simulink model "ofdm_txrx_mimo"  (Simulink 7.1)
settings: Solver=ode45  StartTime=0.0  StopTime=6e3  RelTol=1e-3
PreLoadFcn:
  | ofdm_rx_mimo_init
InitFcn:
  | ofdm_rx_mimo_init
StartFcn:
  | ofdm_rx_mimo_init
values from neighbouring files (symbols this model uses):
  BER_RAM_init_size = 2048    [ofdm_tx_mimo_init.m]
  CPLength = 16    [ofdm_tx_mimo_init.m]
  CRCPolynomial = hex2dec('04c11db7')    [ofdm_tx_mimo_init.m]
  freqOffset_track_filtCoef_i = hex2dec('2500')/2^32    [ofdm_rx_mimo_init.m]
  freqOffset_track_filtCoef_p = hex2dec('d0000')/2^32    [ofdm_rx_mimo_init.m]
  hi = zeros(1,length(cci))    [ofdm_rx_mimo_init.m]
  hr = zeros(1,length(ccr))    [ofdm_rx_mimo_init.m]
  max_CP_length = 16    [ofdm_tx_mimo_init.m]
  max_num_baseRateSymbols = 31    [ofdm_tx_mimo_init.m]
  max_num_subcarriers = 64    [ofdm_tx_mimo_init.m]
  max_num_trainingSymbols = 15    [ofdm_tx_mimo_init.m]
  max_numBytes = 16384    [ofdm_tx_mimo_init.m]
  max_OFDM_symbols = 2047    [ofdm_tx_mimo_init.m]
  modConstellation_qam16 = .75*[1 3 -1 -3]./3    [ofdm_tx_mimo_init.m]
  modConstellation_qam256 = 0.9375*[3 1 5 7 11 9 13 15 -3 -1 -5 -7 -11 -9 -13 -15]./15    [ofdm_tx_mimo_init.m]
  modConstellation_qam64 = 0.875*[3 1 5 7 -3 -1 -5 -7]./7    [ofdm_tx_mimo_init.m]
  modConstellation_qpsk = [1 -1]./sqrt(2)    [ofdm_tx_mimo_init.m]
  numBaseRateSymbols = 2    [ofdm_tx_mimo_init.m]
  numSubcarriers = 64    [ofdm_tx_mimo_init.m]
  numTrainingSymbols = 2    [ofdm_tx_mimo_init.m]
  pilot1_indicies = 7 + ( (64-7) * 2^16)    (= 3735559)    [ofdm_tx_mimo_init.m]
  pilot2_indicies = 21 + ( (64-21) * 2^16)    (= 2818069)    [ofdm_tx_mimo_init.m]
  pilotValue_neg = hex2dec('8000') + (2^16 * 0)    [ofdm_tx_mimo_init.m]
  pilotValue_pos = hex2dec('7FFF') + (2^16 * 0)    [ofdm_tx_mimo_init.m]
  pkt_crossCorr_thresh = 0.7    [ofdm_rx_mimo_init.m]
  pkt_energy_thresh = 0    [ofdm_rx_mimo_init.m]
  pkt_numPayloadBytes = 1400+42-6    (= 1436)    [ofdm_tx_mimo_init.m]
  pktByteNums = numHeaderBytes +  				byteIndex_numPayloadBytes(1)*2^8 +  				byteIndex_numPayloadBytes(2)*2^16 +  				byteIndex_simpleDynModMasks * 2^24    [ofdm_rx_mimo_init.m]
  PktDet_Delay = 26 + Symbol_Timing_Offset*2^7    (= 1050)    [ofdm_rx_mimo_init.m]
  preamble = 6*[0 shortsyms_10 longsyms_2]    [ofdm_tx_mimo_init.m]
  preamble_I = real(preamble)    [ofdm_tx_mimo_init.m]
  preamble_Q = imag(preamble)    [ofdm_tx_mimo_init.m]
  RAM_init_size = max_numBytes    (= 16384)    [ofdm_tx_mimo_init.m]
  reg_Interrupt_PktBuf_Control = reg_InterruptControl +  	1 * 2^16 +  	0 * 2^24    (= 65656)    [ofdm_rx_mimo_init.m]
  rx_controlBits = 1 * 2  		 + 1 * 4  		 + 0 * 8  		 + 1 * 16 * rx_SISO_Mode  		 + 1 * 32  		 + 1 * 64  		 + 0 * 128  		 + 1 * 256  		 + 0 * 512  		 + 1 * 1024  		 + 0 * 2048  		 + 1 * 4096  		 + 1 * 8192  		 + 0 * 16384  		 + 1 * 65536  		 + 1 * 131072  		 + 0 * 262144  		 + 1 * 524288    [ofdm_rx_mimo_init.m]
  Rx_PhaseNoiseTrack_K = 0.6094    [ofdm_rx_mimo_init.m]
  rx_postEq_scaling = round(rxScaling*2^11) * (1 + 2^16)    [ofdm_rx_mimo_init.m]
  RxDataScrambling_Seq = [40 198 78 63 82 173 102 245 48 111 172 115 147 230 216 93 72 65 62 2 205 242 122 90 128 83 105 97 73 10 5 252]    [ofdm_tx_mimo_init.m]
  simOnly_numSamples = length(preamble)+( (numSubcarriers+CPLength)*(numTrainingSymbols + numBaseRateSymbols + numFullRateSymbols/2) )    [ofdm_tx_mimo_init.m]
  train = [train train]    [ofdm_tx_mimo_init.m]
  tx_controlBits = (antB_preambleShift * 2^4) + (2^0 * tx_SISO_Mode) + 4*0 + 8*1    (= 73)    [ofdm_tx_mimo_init.m]
  TxDataScrambling_Seq = [40 198 78 63 82 173 102 245 48 111 172 115 147 230 216 93 72 65 62 2 205 242 122 90 128 83 105 97 73 10 5 252]    [ofdm_tx_mimo_init.m]
  txReg_symbolCounts = (2^16 * numFullRateSymbols) + (2^8 *numBaseRateSymbols ) + numTrainingSymbols    [ofdm_tx_mimo_init.m]
  TxRx_FFTScaling = bin2dec('010111') + (bin2dec('000101') * 2^6)    [ofdm_tx_mimo_init.m]
undefined symbols (not defined in the file or in its folder): AntA, AntB, BaseRate, CRC_table_gen, gcbh, intfilt, pktTiming_count_payloadStart, pktTiming_symbol_length, xlBlockLoadCallback, xlGetSimulinkPeriod
libraries: xbsIndex_r4 x4212, dspmlti4 x4, dspsigops x4, commchan3 x2, simulink x2, commrflib2 x2, dspsrcs4 x1
(part 21 of 43)

== system ofdm_txrx_mimo/OFDM Rx MIMO/Long Corr 4x/C7/C11 ==
  xn : Inport  OutDataType=sfix(16)  OutScaling=2^0
  DAddr : Inport  Port=2  OutDataType=sfix(16)  OutScaling=2^0
  CAddr : Inport  Port=3  OutDataType=sfix(16)  OutScaling=2^0
  LD : Inport  Port=4  OutDataType=sfix(16)  OutScaling=2^0
  Down Sample1 : xbsIndex_r4/Down Sample  sample_ratio=length(coef2)  sample_phase=First Value of Frame  en=off  latency=0  dbl_ovrd=off  block_type=dsamp
      settings: xl_area=[3 3 0 2 0 0 0]  block_version=VER_STRING_GOES_HERE
  Down Sample2 : xbsIndex_r4/Down Sample  sample_ratio=length(coef2)  sample_phase=First Value of Frame  en=off  latency=0  dbl_ovrd=off  block_type=dsamp
      settings: xl_area=[5 5 0 4 0 0 0]  block_version=VER_STRING_GOES_HERE
  MAC : Subsystem
  R : xbsIndex_r4/Addressable Shift Register  infer_latency=off  depth=length(coef2)  initVector=[0]  en=off  dbl_ovrd=off  use_behavioral_HDL=off  use_rpm=off  block_type=addrsr
      settings: infoedit="Delay of  configurable length.  Any element  in the delay     line   can    be   addressed   and   driven    onto   the   output     port.<P><P>Hardware  notes: Implemented  using SRL16s.     If Virtex-4, Virtex-II or Spartan-3 devices are used, multiple SRLC16s are cascaded together."  xl_area=[1 0 0 2 0 0 0]  block_version=VER_STRING_GOES_HERE
  ROM1 : xbsIndex_r4/ROM  depth=length(coef2)  initVector=coef2  distributed_mem=Distributed memory  rst=off  init_reg=0  en=off  latency=1  arith_type=Unsigned  n_bits=1  bin_pt=0  dbl_ovrd=off  optimize=Area  use_rpm=off  block_type=sprom
      settings: xl_area=[1 1 0 1 0 0 0]  block_version=VER_STRING_GOES_HERE
  yn : Outport  OutDataType=sfix(16)  OutScaling=2^0
  xnz : Outport  Port=2  OutDataType=sfix(16)  OutScaling=2^0
  wires:
  Down Sample1/1 -> R/1
  xn/1 -> Down Sample1/1
  DAddr/1 -> R/2
  CAddr/1 -> ROM1/1
  R/1 -> xnz/1, MAC/1(a)
  MAC/1(yn) -> Down Sample2/1
  Down Sample2/1 -> yn/1
  ROM1/1 -> MAC/2(coeff)
  LD/1 -> MAC/3(ld)

== system ofdm_txrx_mimo/OFDM Rx MIMO/Long Corr 4x/C7/C12 ==
  xn : Inport  OutDataType=sfix(16)  OutScaling=2^0
  DAddr : Inport  Port=2  OutDataType=sfix(16)  OutScaling=2^0
  CAddr : Inport  Port=3  OutDataType=sfix(16)  OutScaling=2^0
  LD : Inport  Port=4  OutDataType=sfix(16)  OutScaling=2^0
  Down Sample1 : xbsIndex_r4/Down Sample  sample_ratio=length(coef2)  sample_phase=First Value of Frame  en=off  latency=0  dbl_ovrd=off  block_type=dsamp
      settings: xl_area=[3 3 0 2 0 0 0]  block_version=VER_STRING_GOES_HERE
  Down Sample2 : xbsIndex_r4/Down Sample  sample_ratio=length(coef2)  sample_phase=First Value of Frame  en=off  latency=0  dbl_ovrd=off  block_type=dsamp
      settings: xl_area=[5 5 0 4 0 0 0]  block_version=VER_STRING_GOES_HERE
  MAC : Subsystem
  R : xbsIndex_r4/Addressable Shift Register  infer_latency=off  depth=length(coef2)  initVector=[0]  en=off  dbl_ovrd=off  use_behavioral_HDL=off  use_rpm=off  block_type=addrsr
      settings: infoedit="Delay of  configurable length.  Any element  in the delay     line   can    be   addressed   and   driven    onto   the   output     port.<P><P>Hardware  notes: Implemented  using SRL16s.     If Virtex-4, Virtex-II or Spartan-3 devices are used, multiple SRLC16s are cascaded together."  xl_area=[1 0 0 2 0 0 0]  block_version=VER_STRING_GOES_HERE
  ROM1 : xbsIndex_r4/ROM  depth=length(coef2)  initVector=coef2  distributed_mem=Distributed memory  rst=off  init_reg=0  en=off  latency=1  arith_type=Unsigned  n_bits=1  bin_pt=0  dbl_ovrd=off  optimize=Area  use_rpm=off  block_type=sprom
      settings: xl_area=[1 1 0 1 0 0 0]  block_version=VER_STRING_GOES_HERE
  yn : Outport  OutDataType=sfix(16)  OutScaling=2^0
  xnz : Outport  Port=2  OutDataType=sfix(16)  OutScaling=2^0
  wires:
  Down Sample1/1 -> R/1
  xn/1 -> Down Sample1/1
  DAddr/1 -> R/2
  CAddr/1 -> ROM1/1
  R/1 -> xnz/1, MAC/1(a)
  MAC/1(yn) -> Down Sample2/1
  Down Sample2/1 -> yn/1
  ROM1/1 -> MAC/2(coeff)
  LD/1 -> MAC/3(ld)

== system ofdm_txrx_mimo/OFDM Rx MIMO/Long Corr 4x/C7/C13 ==
  xn : Inport  OutDataType=sfix(16)  OutScaling=2^0
  DAddr : Inport  Port=2  OutDataType=sfix(16)  OutScaling=2^0
  CAddr : Inport  Port=3  OutDataType=sfix(16)  OutScaling=2^0
  LD : Inport  Port=4  OutDataType=sfix(16)  OutScaling=2^0
  Down Sample1 : xbsIndex_r4/Down Sample  sample_ratio=length(coef2)  sample_phase=First Value of Frame  en=off  latency=0  dbl_ovrd=off  block_type=dsamp
      settings: xl_area=[3 3 0 2 0 0 0]  block_version=VER_STRING_GOES_HERE
  Down Sample2 : xbsIndex_r4/Down Sample  sample_ratio=length(coef2)  sample_phase=First Value of Frame  en=off  latency=0  dbl_ovrd=off  block_type=dsamp
      settings: xl_area=[5 5 0 4 0 0 0]  block_version=VER_STRING_GOES_HERE
  MAC : Subsystem
  R : xbsIndex_r4/Addressable Shift Register  infer_latency=off  depth=length(coef2)  initVector=[0]  en=off  dbl_ovrd=off  use_behavioral_HDL=off  use_rpm=off  block_type=addrsr
      settings: infoedit="Delay of  configurable length.  Any element  in the delay     line   can    be   addressed   and   driven    onto   the   output     port.<P><P>Hardware  notes: Implemented  using SRL16s.     If Virtex-4, Virtex-II or Spartan-3 devices are used, multiple SRLC16s are cascaded together."  xl_area=[1 0 0 2 0 0 0]  block_version=VER_STRING_GOES_HERE
  ROM1 : xbsIndex_r4/ROM  depth=length(coef2)  initVector=coef2  distributed_mem=Distributed memory  rst=off  init_reg=0  en=off  latency=1  arith_type=Unsigned  n_bits=1  bin_pt=0  dbl_ovrd=off  optimize=Area  use_rpm=off  block_type=sprom
      settings: xl_area=[1 1 0 1 0 0 0]  block_version=VER_STRING_GOES_HERE
  yn : Outport  OutDataType=sfix(16)  OutScaling=2^0
  xnz : Outport  Port=2  OutDataType=sfix(16)  OutScaling=2^0
  wires:
  Down Sample1/1 -> R/1
  xn/1 -> Down Sample1/1
  DAddr/1 -> R/2
  CAddr/1 -> ROM1/1
  R/1 -> xnz/1, MAC/1(a)
  MAC/1(yn) -> Down Sample2/1
  Down Sample2/1 -> yn/1
  ROM1/1 -> MAC/2(coeff)
  LD/1 -> MAC/3(ld)

== system ofdm_txrx_mimo/OFDM Rx MIMO/Long Corr 4x/C7/C14 ==
  xn : Inport  OutDataType=sfix(16)  OutScaling=2^0
  DAddr : Inport  Port=2  OutDataType=sfix(16)  OutScaling=2^0
  CAddr : Inport  Port=3  OutDataType=sfix(16)  OutScaling=2^0
  LD : Inport  Port=4  OutDataType=sfix(16)  OutScaling=2^0
  Down Sample1 : xbsIndex_r4/Down Sample  sample_ratio=length(coef2)  sample_phase=First Value of Frame  en=off  latency=0  dbl_ovrd=off  block_type=dsamp
      settings: xl_area=[3 3 0 2 0 0 0]  block_version=VER_STRING_GOES_HERE
  Down Sample2 : xbsIndex_r4/Down Sample  sample_ratio=length(coef2)  sample_phase=First Value of Frame  en=off  latency=0  dbl_ovrd=off  block_type=dsamp
      settings: xl_area=[5 5 0 4 0 0 0]  block_version=VER_STRING_GOES_HERE
  MAC : Subsystem
  R : xbsIndex_r4/Addressable Shift Register  infer_latency=off  depth=length(coef2)  initVector=[0]  en=off  dbl_ovrd=off  use_behavioral_HDL=off  use_rpm=off  block_type=addrsr
      settings: infoedit="Delay of  configurable length.  Any element  in the delay     line   can    be   addressed   and   driven    onto   the   output     port.<P><P>Hardware  notes: Implemented  using SRL16s.     If Virtex-4, Virtex-II or Spartan-3 devices are used, multiple SRLC16s are cascaded together."  xl_area=[1 0 0 2 0 0 0]  block_version=VER_STRING_GOES_HERE
  ROM1 : xbsIndex_r4/ROM  depth=length(coef2)  initVector=coef2  distributed_mem=Distributed memory  rst=off  init_reg=0  en=off  latency=1  arith_type=Unsigned  n_bits=1  bin_pt=0  dbl_ovrd=off  optimize=Area  use_rpm=off  block_type=sprom
      settings: xl_area=[1 1 0 1 0 0 0]  block_version=VER_STRING_GOES_HERE
  yn : Outport  OutDataType=sfix(16)  OutScaling=2^0
  xnz : Outport  Port=2  OutDataType=sfix(16)  OutScaling=2^0
  wires:
  LD/1 -> MAC/3(ld)
  ROM1/1 -> MAC/2(coeff)
  Down Sample2/1 -> yn/1
  MAC/1(yn) -> Down Sample2/1
  R/1 -> MAC/1(a), xnz/1
  CAddr/1 -> ROM1/1
  DAddr/1 -> R/2
  xn/1 -> Down Sample1/1
  Down Sample1/1 -> R/1

== system ofdm_txrx_mimo/OFDM Rx MIMO/Long Corr 4x/C7/C15 ==
  xn : Inport  OutDataType=sfix(16)  OutScaling=2^0
  DAddr : Inport  Port=2  OutDataType=sfix(16)  OutScaling=2^0
  CAddr : Inport  Port=3  OutDataType=sfix(16)  OutScaling=2^0
  LD : Inport  Port=4  OutDataType=sfix(16)  OutScaling=2^0
  Down Sample1 : xbsIndex_r4/Down Sample  sample_ratio=length(coef2)  sample_phase=First Value of Frame  en=off  latency=0  dbl_ovrd=off  block_type=dsamp
      settings: xl_area=[3 3 0 2 0 0 0]  block_version=VER_STRING_GOES_HERE
  Down Sample2 : xbsIndex_r4/Down Sample  sample_ratio=length(coef2)  sample_phase=First Value of Frame  en=off  latency=0  dbl_ovrd=off  block_type=dsamp
      settings: xl_area=[5 5 0 4 0 0 0]  block_version=VER_STRING_GOES_HERE
  MAC : Subsystem
  R : xbsIndex_r4/Addressable Shift Register  infer_latency=off  depth=length(coef2)  initVector=[0]  en=off  dbl_ovrd=off  use_behavioral_HDL=off  use_rpm=off  block_type=addrsr
      settings: infoedit="Delay of  configurable length.  Any element  in the delay     line   can    be   addressed   and   driven    onto   the   output     port.<P><P>Hardware  notes: Implemented  using SRL16s.     If Virtex-4, Virtex-II or Spartan-3 devices are used, multiple SRLC16s are cascaded together."  xl_area=[1 0 0 2 0 0 0]  block_version=VER_STRING_GOES_HERE
  ROM1 : xbsIndex_r4/ROM  depth=length(coef2)  initVector=coef2  distributed_mem=Distributed memory  rst=off  init_reg=0  en=off  latency=1  arith_type=Unsigned  n_bits=1  bin_pt=0  dbl_ovrd=off  optimize=Area  use_rpm=off  block_type=sprom
      settings: xl_area=[1 1 0 1 0 0 0]  block_version=VER_STRING_GOES_HERE
  yn : Outport  OutDataType=sfix(16)  OutScaling=2^0
  xnz : Outport  Port=2  OutDataType=sfix(16)  OutScaling=2^0
  wires:
  Down Sample1/1 -> R/1
  xn/1 -> Down Sample1/1
  DAddr/1 -> R/2
  CAddr/1 -> ROM1/1
  R/1 -> xnz/1, MAC/1(a)
  MAC/1(yn) -> Down Sample2/1
  Down Sample2/1 -> yn/1
  ROM1/1 -> MAC/2(coeff)
  LD/1 -> MAC/3(ld)

== system ofdm_txrx_mimo/OFDM Rx MIMO/Long Corr 4x/C7/C16 ==
  xn : Inport  OutDataType=sfix(16)  OutScaling=2^0
  DAddr : Inport  Port=2  OutDataType=sfix(16)  OutScaling=2^0
  CAddr : Inport  Port=3  OutDataType=sfix(16)  OutScaling=2^0
  LD : Inport  Port=4  OutDataType=sfix(16)  OutScaling=2^0
  Down Sample1 : xbsIndex_r4/Down Sample  sample_ratio=length(coef2)  sample_phase=First Value of Frame  en=off  latency=0  dbl_ovrd=off  block_type=dsamp
      settings: xl_area=[3 3 0 2 0 0 0]  block_version=VER_STRING_GOES_HERE
  Down Sample2 : xbsIndex_r4/Down Sample  sample_ratio=length(coef2)  sample_phase=First Value of Frame  en=off  latency=0  dbl_ovrd=off  block_type=dsamp
      settings: xl_area=[5 5 0 4 0 0 0]  block_version=VER_STRING_GOES_HERE
  MAC : Subsystem
  R : xbsIndex_r4/Addressable Shift Register  infer_latency=off  depth=length(coef2)  initVector=[0]  en=off  dbl_ovrd=off  use_behavioral_HDL=off  use_rpm=off  block_type=addrsr
      settings: infoedit="Delay of  configurable length.  Any element  in the delay     line   can    be   addressed   and   driven    onto   the   output     port.<P><P>Hardware  notes: Implemented  using SRL16s.     If Virtex-4, Virtex-II or Spartan-3 devices are used, multiple SRLC16s are cascaded together."  xl_area=[1 0 0 2 0 0 0]  block_version=VER_STRING_GOES_HERE
  ROM1 : xbsIndex_r4/ROM  depth=length(coef2)  initVector=coef2  distributed_mem=Distributed memory  rst=off  init_reg=0  en=off  latency=1  arith_type=Unsigned  n_bits=1  bin_pt=0  dbl_ovrd=off  optimize=Area  use_rpm=off  block_type=sprom
      settings: xl_area=[1 1 0 1 0 0 0]  block_version=VER_STRING_GOES_HERE
  yn : Outport  OutDataType=sfix(16)  OutScaling=2^0
  xnz : Outport  Port=2  OutDataType=sfix(16)  OutScaling=2^0
  wires:
  LD/1 -> MAC/3(ld)
  ROM1/1 -> MAC/2(coeff)
  Down Sample2/1 -> yn/1
  MAC/1(yn) -> Down Sample2/1
  R/1 -> MAC/1(a), xnz/1
  CAddr/1 -> ROM1/1
  DAddr/1 -> R/2
  xn/1 -> Down Sample1/1
  Down Sample1/1 -> R/1

== system ofdm_txrx_mimo/OFDM Rx MIMO/Long Corr 4x/C7/C2 ==
  xn : Inport  OutDataType=sfix(16)  OutScaling=2^0
  DAddr : Inport  Port=2  OutDataType=sfix(16)  OutScaling=2^0
  CAddr : Inport  Port=3  OutDataType=sfix(16)  OutScaling=2^0
  LD : Inport  Port=4  OutDataType=sfix(16)  OutScaling=2^0
  Down Sample1 : xbsIndex_r4/Down Sample  sample_ratio=length(coef2)  sample_phase=First Value of Frame  en=off  latency=0  dbl_ovrd=off  block_type=dsamp
      settings: xl_area=[3 3 0 2 0 0 0]  block_version=VER_STRING_GOES_HERE
  Down Sample2 : xbsIndex_r4/Down Sample  sample_ratio=length(coef2)  sample_phase=First Value of Frame  en=off  latency=0  dbl_ovrd=off  block_type=dsamp
      settings: xl_area=[5 5 0 4 0 0 0]  block_version=VER_STRING_GOES_HERE
  MAC : Subsystem
  R : xbsIndex_r4/Addressable Shift Register  infer_latency=off  depth=length(coef2)  initVector=[0]  en=off  dbl_ovrd=off  use_behavioral_HDL=off  use_rpm=off  block_type=addrsr
      settings: infoedit="Delay of  configurable length.  Any element  in the delay     line   can    be   addressed   and   driven    onto   the   output     port.<P><P>Hardware  notes: Implemented  using SRL16s.     If Virtex-4, Virtex-II or Spartan-3 devices are used, multiple SRLC16s are cascaded together."  xl_area=[1 0 0 2 0 0 0]  block_version=VER_STRING_GOES_HERE
  ROM1 : xbsIndex_r4/ROM  depth=length(coef2)  initVector=coef2  distributed_mem=Distributed memory  rst=off  init_reg=0  en=off  latency=1  arith_type=Unsigned  n_bits=1  bin_pt=0  dbl_ovrd=off  optimize=Area  use_rpm=off  block_type=sprom
      settings: xl_area=[1 1 0 1 0 0 0]  block_version=VER_STRING_GOES_HERE
  yn : Outport  OutDataType=sfix(16)  OutScaling=2^0
  xnz : Outport  Port=2  OutDataType=sfix(16)  OutScaling=2^0
  wires:
  Down Sample1/1 -> R/1
  xn/1 -> Down Sample1/1
  DAddr/1 -> R/2
  CAddr/1 -> ROM1/1
  R/1 -> xnz/1, MAC/1(a)
  MAC/1(yn) -> Down Sample2/1
  Down Sample2/1 -> yn/1
  ROM1/1 -> MAC/2(coeff)
  LD/1 -> MAC/3(ld)

== system ofdm_txrx_mimo/OFDM Rx MIMO/Long Corr 4x/C7/C3 ==
  xn : Inport  OutDataType=sfix(16)  OutScaling=2^0
  DAddr : Inport  Port=2  OutDataType=sfix(16)  OutScaling=2^0
  CAddr : Inport  Port=3  OutDataType=sfix(16)  OutScaling=2^0
  LD : Inport  Port=4  OutDataType=sfix(16)  OutScaling=2^0
  Down Sample1 : xbsIndex_r4/Down Sample  sample_ratio=length(coef2)  sample_phase=First Value of Frame  en=off  latency=0  dbl_ovrd=off  block_type=dsamp
      settings: xl_area=[3 3 0 2 0 0 0]  block_version=VER_STRING_GOES_HERE
  Down Sample2 : xbsIndex_r4/Down Sample  sample_ratio=length(coef2)  sample_phase=First Value of Frame  en=off  latency=0  dbl_ovrd=off  block_type=dsamp
      settings: xl_area=[5 5 0 4 0 0 0]  block_version=VER_STRING_GOES_HERE
  MAC : Subsystem
  R : xbsIndex_r4/Addressable Shift Register  infer_latency=off  depth=length(coef2)  initVector=[0]  en=off  dbl_ovrd=off  use_behavioral_HDL=off  use_rpm=off  block_type=addrsr
      settings: infoedit="Delay of  configurable length.  Any element  in the delay     line   can    be   addressed   and   driven    onto   the   output     port.<P><P>Hardware  notes: Implemented  using SRL16s.     If Virtex-4, Virtex-II or Spartan-3 devices are used, multiple SRLC16s are cascaded together."  xl_area=[1 0 0 2 0 0 0]  block_version=VER_STRING_GOES_HERE
  ROM1 : xbsIndex_r4/ROM  depth=length(coef2)  initVector=coef2  distributed_mem=Distributed memory  rst=off  init_reg=0  en=off  latency=1  arith_type=Unsigned  n_bits=1  bin_pt=0  dbl_ovrd=off  optimize=Area  use_rpm=off  block_type=sprom
      settings: xl_area=[1 1 0 1 0 0 0]  block_version=VER_STRING_GOES_HERE
  yn : Outport  OutDataType=sfix(16)  OutScaling=2^0
  xnz : Outport  Port=2  OutDataType=sfix(16)  OutScaling=2^0
  wires:
  Down Sample1/1 -> R/1
  xn/1 -> Down Sample1/1
  DAddr/1 -> R/2
  CAddr/1 -> ROM1/1
  R/1 -> xnz/1, MAC/1(a)
  MAC/1(yn) -> Down Sample2/1
  Down Sample2/1 -> yn/1
  ROM1/1 -> MAC/2(coeff)
  LD/1 -> MAC/3(ld)

== system ofdm_txrx_mimo/OFDM Rx MIMO/Long Corr 4x/C7/C4 ==
  xn : Inport  OutDataType=sfix(16)  OutScaling=2^0
  DAddr : Inport  Port=2  OutDataType=sfix(16)  OutScaling=2^0
  CAddr : Inport  Port=3  OutDataType=sfix(16)  OutScaling=2^0
  LD : Inport  Port=4  OutDataType=sfix(16)  OutScaling=2^0
  Down Sample1 : xbsIndex_r4/Down Sample  sample_ratio=length(coef2)  sample_phase=First Value of Frame  en=off  latency=0  dbl_ovrd=off  block_type=dsamp
      settings: xl_area=[3 3 0 2 0 0 0]  block_version=VER_STRING_GOES_HERE
  Down Sample2 : xbsIndex_r4/Down Sample  sample_ratio=length(coef2)  sample_phase=First Value of Frame  en=off  latency=0  dbl_ovrd=off  block_type=dsamp
      settings: xl_area=[5 5 0 4 0 0 0]  block_version=VER_STRING_GOES_HERE
  MAC : Subsystem
  R : xbsIndex_r4/Addressable Shift Register  infer_latency=off  depth=length(coef2)  initVector=[0]  en=off  dbl_ovrd=off  use_behavioral_HDL=off  use_rpm=off  block_type=addrsr
      settings: infoedit="Delay of  configurable length.  Any element  in the delay     line   can    be   addressed   and   driven    onto   the   output     port.<P><P>Hardware  notes: Implemented  using SRL16s.     If Virtex-4, Virtex-II or Spartan-3 devices are used, multiple SRLC16s are cascaded together."  xl_area=[1 0 0 2 0 0 0]  block_version=VER_STRING_GOES_HERE
  ROM1 : xbsIndex_r4/ROM  depth=length(coef2)  initVector=coef2  distributed_mem=Distributed memory  rst=off  init_reg=0  en=off  latency=1  arith_type=Unsigned  n_bits=1  bin_pt=0  dbl_ovrd=off  optimize=Area  use_rpm=off  block_type=sprom
      settings: xl_area=[1 1 0 1 0 0 0]  block_version=VER_STRING_GOES_HERE
  yn : Outport  OutDataType=sfix(16)  OutScaling=2^0
  xnz : Outport  Port=2  OutDataType=sfix(16)  OutScaling=2^0
  wires:
  Down Sample1/1 -> R/1
  xn/1 -> Down Sample1/1
  DAddr/1 -> R/2
  CAddr/1 -> ROM1/1
  R/1 -> xnz/1, MAC/1(a)
  MAC/1(yn) -> Down Sample2/1
  Down Sample2/1 -> yn/1
  ROM1/1 -> MAC/2(coeff)
  LD/1 -> MAC/3(ld)

== system ofdm_txrx_mimo/OFDM Rx MIMO/Long Corr 4x/C7/C5 ==
  xn : Inport  OutDataType=sfix(16)  OutScaling=2^0
  DAddr : Inport  Port=2  OutDataType=sfix(16)  OutScaling=2^0
  CAddr : Inport  Port=3  OutDataType=sfix(16)  OutScaling=2^0
  LD : Inport  Port=4  OutDataType=sfix(16)  OutScaling=2^0
  Down Sample1 : xbsIndex_r4/Down Sample  sample_ratio=length(coef2)  sample_phase=First Value of Frame  en=off  latency=0  dbl_ovrd=off  block_type=dsamp
      settings: xl_area=[3 3 0 2 0 0 0]  block_version=VER_STRING_GOES_HERE
  Down Sample2 : xbsIndex_r4/Down Sample  sample_ratio=length(coef2)  sample_phase=First Value of Frame  en=off  latency=0  dbl_ovrd=off  block_type=dsamp
      settings: xl_area=[5 5 0 4 0 0 0]  block_version=VER_STRING_GOES_HERE
  MAC : Subsystem
  R : xbsIndex_r4/Addressable Shift Register  infer_latency=off  depth=length(coef2)  initVector=[0]  en=off  dbl_ovrd=off  use_behavioral_HDL=off  use_rpm=off  block_type=addrsr
      settings: infoedit="Delay of  configurable length.  Any element  in the delay     line   can    be   addressed   and   driven    onto   the   output     port.<P><P>Hardware  notes: Implemented  using SRL16s.     If Virtex-4, Virtex-II or Spartan-3 devices are used, multiple SRLC16s are cascaded together."  xl_area=[1 0 0 2 0 0 0]  block_version=VER_STRING_GOES_HERE
  ROM1 : xbsIndex_r4/ROM  depth=length(coef2)  initVector=coef2  distributed_mem=Distributed memory  rst=off  init_reg=0  en=off  latency=1  arith_type=Unsigned  n_bits=1  bin_pt=0  dbl_ovrd=off  optimize=Area  use_rpm=off  block_type=sprom
      settings: xl_area=[1 1 0 1 0 0 0]  block_version=VER_STRING_GOES_HERE
  yn : Outport  OutDataType=sfix(16)  OutScaling=2^0
  xnz : Outport  Port=2  OutDataType=sfix(16)  OutScaling=2^0
  wires:
  Down Sample1/1 -> R/1
  xn/1 -> Down Sample1/1
  DAddr/1 -> R/2
  CAddr/1 -> ROM1/1
  R/1 -> xnz/1, MAC/1(a)
  MAC/1(yn) -> Down Sample2/1
  Down Sample2/1 -> yn/1
  ROM1/1 -> MAC/2(coeff)
  LD/1 -> MAC/3(ld)

== system ofdm_txrx_mimo/OFDM Rx MIMO/Long Corr 4x/C7/C6 ==
  xn : Inport  OutDataType=sfix(16)  OutScaling=2^0
  DAddr : Inport  Port=2  OutDataType=sfix(16)  OutScaling=2^0
  CAddr : Inport  Port=3  OutDataType=sfix(16)  OutScaling=2^0
  LD : Inport  Port=4  OutDataType=sfix(16)  OutScaling=2^0
  Down Sample1 : xbsIndex_r4/Down Sample  sample_ratio=length(coef2)  sample_phase=First Value of Frame  en=off  latency=0  dbl_ovrd=off  block_type=dsamp
      settings: xl_area=[3 3 0 2 0 0 0]  block_version=VER_STRING_GOES_HERE
  Down Sample2 : xbsIndex_r4/Down Sample  sample_ratio=length(coef2)  sample_phase=First Value of Frame  en=off  latency=0  dbl_ovrd=off  block_type=dsamp
      settings: xl_area=[5 5 0 4 0 0 0]  block_version=VER_STRING_GOES_HERE
  MAC : Subsystem
  R : xbsIndex_r4/Addressable Shift Register  infer_latency=off  depth=length(coef2)  initVector=[0]  en=off  dbl_ovrd=off  use_behavioral_HDL=off  use_rpm=off  block_type=addrsr
      settings: infoedit="Delay of  configurable length.  Any element  in the delay     line   can    be   addressed   and   driven    onto   the   output     port.<P><P>Hardware  notes: Implemented  using SRL16s.     If Virtex-4, Virtex-II or Spartan-3 devices are used, multiple SRLC16s are cascaded together."  xl_area=[1 0 0 2 0 0 0]  block_version=VER_STRING_GOES_HERE
  ROM1 : xbsIndex_r4/ROM  depth=length(coef2)  initVector=coef2  distributed_mem=Distributed memory  rst=off  init_reg=0  en=off  latency=1  arith_type=Unsigned  n_bits=1  bin_pt=0  dbl_ovrd=off  optimize=Area  use_rpm=off  block_type=sprom
      settings: xl_area=[1 1 0 1 0 0 0]  block_version=VER_STRING_GOES_HERE
  yn : Outport  OutDataType=sfix(16)  OutScaling=2^0
  xnz : Outport  Port=2  OutDataType=sfix(16)  OutScaling=2^0
  wires:
  Down Sample1/1 -> R/1
  xn/1 -> Down Sample1/1
  DAddr/1 -> R/2
  CAddr/1 -> ROM1/1
  R/1 -> xnz/1, MAC/1(a)
  MAC/1(yn) -> Down Sample2/1
  Down Sample2/1 -> yn/1
  ROM1/1 -> MAC/2(coeff)
  LD/1 -> MAC/3(ld)

== system ofdm_txrx_mimo/OFDM Rx MIMO/Long Corr 4x/C7/C7 ==
  xn : Inport  OutDataType=sfix(16)  OutScaling=2^0
  DAddr : Inport  Port=2  OutDataType=sfix(16)  OutScaling=2^0
  CAddr : Inport  Port=3  OutDataType=sfix(16)  OutScaling=2^0
  LD : Inport  Port=4  OutDataType=sfix(16)  OutScaling=2^0
  Down Sample1 : xbsIndex_r4/Down Sample  sample_ratio=length(coef2)  sample_phase=First Value of Frame  en=off  latency=0  dbl_ovrd=off  block_type=dsamp
      settings: xl_area=[3 3 0 2 0 0 0]  block_version=VER_STRING_GOES_HERE
  Down Sample2 : xbsIndex_r4/Down Sample  sample_ratio=length(coef2)  sample_phase=First Value of Frame  en=off  latency=0  dbl_ovrd=off  block_type=dsamp
      settings: xl_area=[5 5 0 4 0 0 0]  block_version=VER_STRING_GOES_HERE
  MAC : Subsystem
  R : xbsIndex_r4/Addressable Shift Register  infer_latency=off  depth=length(coef2)  initVector=[0]  en=off  dbl_ovrd=off  use_behavioral_HDL=off  use_rpm=off  block_type=addrsr
      settings: infoedit="Delay of  configurable length.  Any element  in the delay     line   can    be   addressed   and   driven    onto   the   output     port.<P><P>Hardware  notes: Implemented  using SRL16s.     If Virtex-4, Virtex-II or Spartan-3 devices are used, multiple SRLC16s are cascaded together."  xl_area=[1 0 0 2 0 0 0]  block_version=VER_STRING_GOES_HERE
  ROM1 : xbsIndex_r4/ROM  depth=length(coef2)  initVector=coef2  distributed_mem=Distributed memory  rst=off  init_reg=0  en=off  latency=1  arith_type=Unsigned  n_bits=1  bin_pt=0  dbl_ovrd=off  optimize=Area  use_rpm=off  block_type=sprom
      settings: xl_area=[1 1 0 1 0 0 0]  block_version=VER_STRING_GOES_HERE
  yn : Outport  OutDataType=sfix(16)  OutScaling=2^0
  xnz : Outport  Port=2  OutDataType=sfix(16)  OutScaling=2^0
  wires:
  Down Sample1/1 -> R/1
  xn/1 -> Down Sample1/1
  DAddr/1 -> R/2
  CAddr/1 -> ROM1/1
  R/1 -> xnz/1, MAC/1(a)
  MAC/1(yn) -> Down Sample2/1
  Down Sample2/1 -> yn/1
  ROM1/1 -> MAC/2(coeff)
  LD/1 -> MAC/3(ld)

== system ofdm_txrx_mimo/OFDM Rx MIMO/Long Corr 4x/C7/C8 ==
  xn : Inport  OutDataType=sfix(16)  OutScaling=2^0
  DAddr : Inport  Port=2  OutDataType=sfix(16)  OutScaling=2^0
  CAddr : Inport  Port=3  OutDataType=sfix(16)  OutScaling=2^0
  LD : Inport  Port=4  OutDataType=sfix(16)  OutScaling=2^0
  Down Sample1 : xbsIndex_r4/Down Sample  sample_ratio=length(coef2)  sample_phase=First Value of Frame  en=off  latency=0  dbl_ovrd=off  block_type=dsamp
      settings: xl_area=[3 3 0 2 0 0 0]  block_version=VER_STRING_GOES_HERE
  Down Sample2 : xbsIndex_r4/Down Sample  sample_ratio=length(coef2)  sample_phase=First Value of Frame  en=off  latency=0  dbl_ovrd=off  block_type=dsamp
      settings: xl_area=[5 5 0 4 0 0 0]  block_version=VER_STRING_GOES_HERE
  MAC : Subsystem
  R : xbsIndex_r4/Addressable Shift Register  infer_latency=off  depth=length(coef2)  initVector=[0]  en=off  dbl_ovrd=off  use_behavioral_HDL=off  use_rpm=off  block_type=addrsr
      settings: infoedit="Delay of  configurable length.  Any element  in the delay     line   can    be   addressed   and   driven    onto   the   output     port.<P><P>Hardware  notes: Implemented  using SRL16s.     If Virtex-4, Virtex-II or Spartan-3 devices are used, multiple SRLC16s are cascaded together."  xl_area=[1 0 0 2 0 0 0]  block_version=VER_STRING_GOES_HERE
  ROM1 : xbsIndex_r4/ROM  depth=length(coef2)  initVector=coef2  distributed_mem=Distributed memory  rst=off  init_reg=0  en=off  latency=1  arith_type=Unsigned  n_bits=1  bin_pt=0  dbl_ovrd=off  optimize=Area  use_rpm=off  block_type=sprom
      settings: xl_area=[1 1 0 1 0 0 0]  block_version=VER_STRING_GOES_HERE
  yn : Outport  OutDataType=sfix(16)  OutScaling=2^0
  xnz : Outport  Port=2  OutDataType=sfix(16)  OutScaling=2^0
  wires:
  Down Sample1/1 -> R/1
  xn/1 -> Down Sample1/1
  DAddr/1 -> R/2
  CAddr/1 -> ROM1/1
  R/1 -> xnz/1, MAC/1(a)
  MAC/1(yn) -> Down Sample2/1
  Down Sample2/1 -> yn/1
  ROM1/1 -> MAC/2(coeff)
  LD/1 -> MAC/3(ld)

== system ofdm_txrx_mimo/OFDM Rx MIMO/Long Corr 4x/C7/C9 ==
  xn : Inport  OutDataType=sfix(16)  OutScaling=2^0
  DAddr : Inport  Port=2  OutDataType=sfix(16)  OutScaling=2^0
  CAddr : Inport  Port=3  OutDataType=sfix(16)  OutScaling=2^0
  LD : Inport  Port=4  OutDataType=sfix(16)  OutScaling=2^0
  Down Sample1 : xbsIndex_r4/Down Sample  sample_ratio=length(coef2)  sample_phase=First Value of Frame  en=off  latency=0  dbl_ovrd=off  block_type=dsamp
      settings: xl_area=[3 3 0 2 0 0 0]  block_version=VER_STRING_GOES_HERE
  Down Sample2 : xbsIndex_r4/Down Sample  sample_ratio=length(coef2)  sample_phase=First Value of Frame  en=off  latency=0  dbl_ovrd=off  block_type=dsamp
      settings: xl_area=[5 5 0 4 0 0 0]  block_version=VER_STRING_GOES_HERE
  MAC : Subsystem
  R : xbsIndex_r4/Addressable Shift Register  infer_latency=off  depth=length(coef2)  initVector=[0]  en=off  dbl_ovrd=off  use_behavioral_HDL=off  use_rpm=off  block_type=addrsr
      settings: infoedit="Delay of  configurable length.  Any element  in the delay     line   can    be   addressed   and   driven    onto   the   output     port.<P><P>Hardware  notes: Implemented  using SRL16s.     If Virtex-4, Virtex-II or Spartan-3 devices are used, multiple SRLC16s are cascaded together."  xl_area=[1 0 0 2 0 0 0]  block_version=VER_STRING_GOES_HERE
  ROM1 : xbsIndex_r4/ROM  depth=length(coef2)  initVector=coef2  distributed_mem=Distributed memory  rst=off  init_reg=0  en=off  latency=1  arith_type=Unsigned  n_bits=1  bin_pt=0  dbl_ovrd=off  optimize=Area  use_rpm=off  block_type=sprom
      settings: xl_area=[1 1 0 1 0 0 0]  block_version=VER_STRING_GOES_HERE
  yn : Outport  OutDataType=sfix(16)  OutScaling=2^0
  xnz : Outport  Port=2  OutDataType=sfix(16)  OutScaling=2^0
  wires:
  Down Sample1/1 -> R/1
  xn/1 -> Down Sample1/1
  DAddr/1 -> R/2
  CAddr/1 -> ROM1/1
  R/1 -> xnz/1, MAC/1(a)
  MAC/1(yn) -> Down Sample2/1
  Down Sample2/1 -> yn/1
  ROM1/1 -> MAC/2(coeff)
  LD/1 -> MAC/3(ld)

== system ofdm_txrx_mimo/OFDM Rx MIMO/Long Corr 4x/C7/FSM ==
  Coef Addr Gen : xbsIndex_r4/Counter  cnt_type=Free Running  cnt_to=Inf  operation=Up  start_count=0  cnt_by_val=1  arith_type=Unsigned  n_bits=ceil(log2(length(h(1:N))))  bin_pt=0  load_pin=off  rst=on  en=off  explicit_period=off  period=1  dbl_ovrd=off  use_behavioral_HDL=off  use_rpm=on  implementation=Fabric  block_type=counter
      settings: xl_area=[2 3 0 3 0 0 0]  block_version=VER_STRING_GOES_HERE
  Constant2 : xbsIndex_r4/Constant  arith_type=Unsigned  const=0  n_bits=ceil(log2(length(h(1:N))/2))+1  bin_pt=0  explicit_period=off  period=2/N  equ=P=C  opselect=C  inp2=PCIN>>17  opr=+  inp1=P  carry=CIN  dbl_ovrd=off  block_type=constant
      settings: block_version=VER_STRING_GOES_HERE
  Constant3 : xbsIndex_r4/Constant  arith_type=Unsigned  const=length(h(1:N))-1  n_bits=ceil(log2(length(h(1:N))))  bin_pt=0  explicit_period=off  period=2/N  equ=P=C  opselect=C  inp2=PCIN>>17  opr=+  inp1=P  carry=CIN  dbl_ovrd=off  block_type=constant
      settings: block_version=VER_STRING_GOES_HERE
  Data Addr Gen : xbsIndex_r4/Counter  cnt_type=Count Limited  cnt_to=length(h(1:N))-1  operation=Up  start_count=0  cnt_by_val=1  arith_type=Unsigned  n_bits=ceil(log2(length(h(1:N))))  bin_pt=0  load_pin=off  rst=off  en=off  explicit_period=on  period=Ts_In/N  dbl_ovrd=off  use_behavioral_HDL=off  use_rpm=on  implementation=Fabric  block_type=counter
      settings: xl_area=[3 3 0 4 0 0 0]  block_version=VER_STRING_GOES_HERE
  Delay1 : xbsIndex_r4/Delay  en=off  latency=1  dbl_ovrd=off  reg_retiming=off  block_type=delay
      settings: infoedit=Hardware notes: A delay line is a chain, each link of which is an SRL16 followed by a flip-flop. If register retiming is enabled, the delay line is a chain of flip-flops.  xl_area=[2 3 0 0 0 0 0]  block_version=VER_STRING_GOES_HERE
  Gateway Out : xbsIndex_r4/Gateway Out  hdl_port=off  timing_constraint=None  locs_specified=off  LOCs={}  block_type=gatewayout
      settings: infoedit="Gateway out block.  Converts Xilinx fixed point inputs into ouputs of type Simulink integer, double, or fixed point.<P><P>Hardware notes:  In hardware these blocks become top level output ports or are discarded, depending on how they are configured."  xl_area=[0 0 0 0 0 0 0]  block_version=VER_STRING_GOES_HERE
  Gateway Out1 : xbsIndex_r4/Gateway Out  hdl_port=off  timing_constraint=None  locs_specified=off  LOCs={}  block_type=gatewayout
      settings: infoedit="Gateway out block.  Converts Xilinx fixed point inputs into ouputs of type Simulink integer, double, or fixed point.<P><P>Hardware notes:  In hardware these blocks become top level output ports or are discarded, depending on how they are configured."  xl_area=[0 0 0 0 0 0 0]  block_version=VER_STRING_GOES_HERE
  Gateway Out3 : xbsIndex_r4/Gateway Out  hdl_port=off  timing_constraint=None  locs_specified=off  LOCs={}  block_type=gatewayout
      settings: infoedit="Gateway out block.  Converts Xilinx fixed point inputs into ouputs of type Simulink integer, double, or fixed point.<P><P>Hardware notes:  In hardware these blocks become top level output ports or are discarded, depending on how they are configured."  xl_area=[0 0 0 0 0 0 0]  block_version=VER_STRING_GOES_HERE
  Long Corr Scope : Scope  NumInputPorts=3
      settings: YMin=-1~-1~-35  YMax=1~1~25  SaveName=ScopeData4
  Relational2 : xbsIndex_r4/Relational  mode=a=b  en=off  latency=1  dbl_ovrd=off  block_type=relational
      settings: xl_area=[1 1 0 2 0 0 0]  block_version=VER_STRING_GOES_HERE
  Relational3 : xbsIndex_r4/Relational  mode=a=b  en=off  latency=1  dbl_ovrd=off  block_type=relational
      settings: xl_area=[1 1 0 2 0 0 0]  block_version=VER_STRING_GOES_HERE
  bc3 : xbsIndex_r4/Convert  arith_type=Unsigned  n_bits=1  bin_pt=0  quantization=Truncate  overflow=Wrap  latency=0  dbl_ovrd=off  pipeline=off  block_type=convert
      settings: xl_area=[0 0 0 0 0 0 0]  block_version=VER_STRING_GOES_HERE
  Data Addr : Outport  OutDataType=sfix(16)  OutScaling=2^0
  Coef Addr : Outport  Port=2  OutDataType=sfix(16)  OutScaling=2^0
  load : Outport  Port=3  OutDataType=sfix(16)  OutScaling=2^0
  wires:
  bc3/1 -> load/1
  Relational3/1 -> bc3/1, Gateway Out3/1
  Constant3/1 -> Relational3/2
  Delay1/1 -> Coef Addr/1, Relational3/1
  Constant2/1 -> Relational2/2
  Relational2/1 -> Coef Addr Gen/1
  Coef Addr Gen/1 -> Delay1/1, Gateway Out1/1
  Data Addr Gen/1 -> Relational2/1, Data Addr/1, Gateway Out/1
  Gateway Out/1 -> Long Corr Scope/1
  Gateway Out1/1 -> Long Corr Scope/2
  Gateway Out3/1 -> Long Corr Scope/3

== system ofdm_txrx_mimo/OFDM Rx MIMO/Packet_Detection/Internal PktDet (Sim Only!)/A*conj(B) 4xCLK ==
  Re[A] : Inport  OutDataType=sfix(16)  OutScaling=2^0
  Re[B] : Inport  Port=2  OutDataType=sfix(16)  OutScaling=2^0
  Im[A] : Inport  Port=3  OutDataType=sfix(16)  OutScaling=2^0
  Im[B] : Inport  Port=4  OutDataType=sfix(16)  OutScaling=2^0
  Add1 : xbsIndex_r4/AddSub  mode=Addition  use_carryin=off  use_carryout=off  en=off  latency=0  precision=User Defined  arith_type="Signed  (2's comp)"  n_bits=17  bin_pt=15  quantization=Truncate  overflow=Flag as error  dbl_ovrd=off  use_behavioral_HDL=on  pipelined=off  use_rpm=on  hw_selection=Fabric  block_type=addsub
      settings: xl_area=[9 0 0 16 0 0 0]  block_version=VER_STRING_GOES_HERE
  Counter : xbsIndex_r4/Counter  Orientation=down  cnt_type=Free Running  cnt_to=4  operation=Up  start_count=0  cnt_by_val=1  arith_type=Unsigned  n_bits=2  bin_pt=0  load_pin=off  rst=off  en=off  explicit_period=on  period=1  dbl_ovrd=off  use_behavioral_HDL=off  use_rpm=on  implementation=Fabric  block_type=counter
      settings: xl_area=[3 3 0 4 0 0 0]  block_version=VER_STRING_GOES_HERE
  Delay1 : xbsIndex_r4/Delay  en=off  latency=1  dbl_ovrd=off  reg_retiming=off  block_type=delay
      settings: infoedit=Hardware notes: A delay line is a chain, each link of which is an SRL16 followed by a flip-flop. If register retiming is enabled, the delay line is a chain of flip-flops.  xl_area=[8 16 0 0 0 0 0]  block_version=VER_STRING_GOES_HERE
  Delay2 : xbsIndex_r4/Delay  en=off  latency=2  dbl_ovrd=off  reg_retiming=off  block_type=delay
      settings: infoedit=Hardware notes: A delay line is a chain, each link of which is an SRL16 followed by a flip-flop. If register retiming is enabled, the delay line is a chain of flip-flops.  xl_area=[9 17 0 17 0 0 0]  block_version=VER_STRING_GOES_HERE
  Delay3 : xbsIndex_r4/Delay  en=off  latency=0  dbl_ovrd=off  reg_retiming=off  block_type=delay
      settings: infoedit=Hardware notes: A delay line is a chain, each link of which is an SRL16 followed by a flip-flop. If register retiming is enabled, the delay line is a chain of flip-flops.  xl_area=[9 17 0 17 0 0 0]  block_version=VER_STRING_GOES_HERE
  Down Sample3 : xbsIndex_r4/Down Sample  sample_ratio=4  sample_phase=First Value of Frame  en=off  latency=1  dbl_ovrd=off  block_type=dsamp
      settings: xl_area=[9 17 0 0 0 0 0]  block_version=VER_STRING_GOES_HERE
  Down Sample4 : xbsIndex_r4/Down Sample  sample_ratio=4  sample_phase=First Value of Frame  en=off  latency=1  dbl_ovrd=off  block_type=dsamp
      settings: xl_area=[9 17 0 0 0 0 0]  block_version=VER_STRING_GOES_HERE
  Mult4 : xbsIndex_r4/Mult  precision=User Defined  arith_type="Signed  (2's comp)"  n_bits=16  bin_pt=15  quantization=Truncate  overflow=Flag as error  en=off  latency=1  dbl_ovrd=off  use_behavioral_HDL=off  use_embedded=on  opt=Speed  optimum_pipeline=off  pipeline=on  use_rpm=off  placement_style=Rectangular shape  block_type=mult
      settings: infoedit=Hardware notes: To use the internal pipeline stage of the dedicated multiplier you must select 'Pipeline for maximum performance'.  xl_area=[14 27 0 27 0 1 0]  block_version=VER_STRING_GOES_HERE
  Mux : xbsIndex_r4/Mux  inputs=4  en=off  latency=0  precision=Full  arith_type="Signed  (2's comp)"  n_bits=16  bin_pt=13  quantization=Truncate  overflow=Wrap  dbl_ovrd=off  block_type=mux
      settings: xl_area=[14 0 0 28 0 0 0]  block_version=VER_STRING_GOES_HERE
  Mux1 : xbsIndex_r4/Mux  inputs=4  en=off  latency=0  precision=Full  arith_type="Signed  (2's comp)"  n_bits=16  bin_pt=13  quantization=Truncate  overflow=Wrap  dbl_ovrd=off  block_type=mux
      settings: xl_area=[14 0 0 28 0 0 0]  block_version=VER_STRING_GOES_HERE
  Negate : xbsIndex_r4/Negate  precision=User Defined  arith_type="Signed  (2's comp)"  n_bits=14  bin_pt=13  quantization=Truncate  overflow=Wrap  en=off  latency=0  dbl_ovrd=off  block_type=negate
      settings: xl_area=[7 0 0 14 0 0 0]  block_version=VER_STRING_GOES_HERE
  Negate1 : xbsIndex_r4/Negate  precision=User Defined  arith_type="Signed  (2's comp)"  n_bits=14  bin_pt=13  quantization=Truncate  overflow=Wrap  en=off  latency=0  dbl_ovrd=off  block_type=negate
      settings: xl_area=[7 0 0 14 0 0 0]  block_version=VER_STRING_GOES_HERE
  Slice : xbsIndex_r4/Slice  nbits=2  boolean_output=off  mode=Lower Bit Location + Width  bit1=0  base1=MSB of Input  bit0=0  base0=LSB of Input  dbl_ovrd=off  block_type=slice
      settings: infoedit="Extracts a given range of bits from each input sample and presents it at the output.  The output type is ordinarily unsigned with binary point at zero, but can be Boolean when the slice is one bit wide.<P><P>Hardware notes: In hardware this block costs nothing."  block_version=VER_STRING_GOES_HERE
  Up Sample{1..4} (4 alike) : xbsIndex_r4/Up Sample  sample_ratio=4  copy_samples=on  dbl_ovrd=off  block_type=usamp
      settings: infoedit="Up samples input data.  Inserted values  can be zeros or copies of the most recent input sample.<P><P>Hardware notes: No hardware is needed if inserted values are copies of the input sample; otherwise, a mux and single bit flip-flop are used."  xl_area=[0 0 0 0 0 0 0]  block_version=VER_STRING_GOES_HERE
  Re : Outport  OutDataType=sfix(16)  OutScaling=2^0
  Im1 : Outport  Port=2  OutDataType=sfix(16)  OutScaling=2^0
  wires:
  Delay3/1 -> Down Sample4/1
  Slice/1 -> Mux/1, Mux1/1
  Counter/1 -> Slice/1
  Delay1/1 -> Add1/2
  Mult4/1 -> Delay1/1, Add1/1
  Up Sample1/1 -> Mux1/5, Negate/1
  Up Sample4/1 -> Negate1/1
  Up Sample2/1 -> Mux/2, Mux/5
  Up Sample3/1 -> Mux1/4, Mux1/2
  Re[A]/1 -> Up Sample3/1
  Re[B]/1 -> Up Sample2/1
  Im[A]/1 -> Up Sample1/1
  Im[B]/1 -> Up Sample4/1
  Mux1/1 -> Mult4/1
  Mux/1 -> Mult4/2
  Delay2/1 -> Down Sample3/1
  Down Sample4/1 -> Im1/1
  Down Sample3/1 -> Re/1
  Add1/1 -> Delay2/1, Delay3/1
  Negate1/1 -> Mux/4, Mux/3
  Negate/1 -> Mux1/3

== system ofdm_txrx_mimo/OFDM Rx MIMO/Packet_Detection/Internal PktDet (Sim Only!)/Magnitude Squared ==
  I : Inport  OutDataType=sfix(16)  OutScaling=2^0
  Q : Inport  Port=2  OutDataType=sfix(16)  OutScaling=2^0
  AddSub1 : xbsIndex_r4/AddSub  mode=Addition  use_carryin=off  use_carryout=off  en=off  latency=addLatency  precision=User Defined  arith_type=Unsigned  n_bits=prec  bin_pt=bp  quantization=Truncate  overflow=Flag as error  dbl_ovrd=off  use_behavioral_HDL=on  pipelined=off  use_rpm=on  hw_selection=Fabric  block_type=addsub
      settings: xl_area=[12 22 0 22 0 0 0]  block_version=VER_STRING_GOES_HERE
  Mult1 : xbsIndex_r4/Mult  precision=User Defined  arith_type="Signed  (2's comp)"  n_bits=prec  bin_pt=bp+1  quantization=Truncate  overflow=Flag as error  en=off  latency=multLatency  dbl_ovrd=off  use_behavioral_HDL=off  use_embedded=on  opt=Speed  optimum_pipeline=off  pipeline=on  use_rpm=off  placement_style=Rectangular shape  block_type=mult
      settings: infoedit=Hardware notes: To use the internal pipeline stage of the dedicated multiplier you must select 'Pipeline for maximum performance'.  xl_area=[63 105 0 106 0 4 0]  block_version=VER_STRING_GOES_HERE
  Mult4 : xbsIndex_r4/Mult  precision=User Defined  arith_type="Signed  (2's comp)"  n_bits=prec  bin_pt=bp+1  quantization=Truncate  overflow=Flag as error  en=off  latency=multLatency  dbl_ovrd=off  use_behavioral_HDL=off  use_embedded=on  opt=Speed  optimum_pipeline=off  pipeline=on  use_rpm=off  placement_style=Rectangular shape  block_type=mult
      settings: infoedit=Hardware notes: To use the internal pipeline stage of the dedicated multiplier you must select 'Pipeline for maximum performance'.  xl_area=[63 105 0 106 0 4 0]  block_version=VER_STRING_GOES_HERE
  Mag : Outport  OutDataType=sfix(16)  OutScaling=2^0
  wires:
  AddSub1/1 -> Mag/1
  Mult1/1 -> AddSub1/2
  Mult4/1 -> AddSub1/1
  Q/1 -> Mult1/2, Mult1/1
  I/1 -> Mult4/2, Mult4/1

== system ofdm_txrx_mimo/OFDM Rx MIMO/Packet_Detection/Internal PktDet (Sim Only!)/Magnitude Squared1 ==
  I : Inport  OutDataType=sfix(16)  OutScaling=2^0
  Q : Inport  Port=2  OutDataType=sfix(16)  OutScaling=2^0
  AddSub1 : xbsIndex_r4/AddSub  mode=Addition  use_carryin=off  use_carryout=off  en=off  latency=addLatency  precision=User Defined  arith_type=Unsigned  n_bits=prec  bin_pt=bp  quantization=Truncate  overflow=Flag as error  dbl_ovrd=off  use_behavioral_HDL=on  pipelined=off  use_rpm=on  hw_selection=Fabric  block_type=addsub
      settings: xl_area=[9 0 0 17 0 0 0]  block_version=VER_STRING_GOES_HERE
  Mult1 : xbsIndex_r4/Mult  precision=User Defined  arith_type="Signed  (2's comp)"  n_bits=prec  bin_pt=bp+1  quantization=Truncate  overflow=Flag as error  en=off  latency=multLatency  dbl_ovrd=off  use_behavioral_HDL=off  use_embedded=on  opt=Speed  optimum_pipeline=off  pipeline=on  use_rpm=off  placement_style=Rectangular shape  block_type=mult
      settings: infoedit=Hardware notes: To use the internal pipeline stage of the dedicated multiplier you must select 'Pipeline for maximum performance'.  xl_area=[9 17 0 17 0 1 0]  block_version=VER_STRING_GOES_HERE
  Mult4 : xbsIndex_r4/Mult  precision=User Defined  arith_type="Signed  (2's comp)"  n_bits=prec  bin_pt=bp+1  quantization=Truncate  overflow=Flag as error  en=off  latency=multLatency  dbl_ovrd=off  use_behavioral_HDL=off  use_embedded=on  opt=Speed  optimum_pipeline=off  pipeline=on  use_rpm=off  placement_style=Rectangular shape  block_type=mult
      settings: infoedit=Hardware notes: To use the internal pipeline stage of the dedicated multiplier you must select 'Pipeline for maximum performance'.  xl_area=[9 17 0 17 0 1 0]  block_version=VER_STRING_GOES_HERE
  Mag : Outport  OutDataType=sfix(16)  OutScaling=2^0
  wires:
  I/1 -> Mult4/1, Mult4/2
  Q/1 -> Mult1/1, Mult1/2
  Mult4/1 -> AddSub1/1
  Mult1/1 -> AddSub1/2
  AddSub1/1 -> Mag/1

== system ofdm_txrx_mimo/OFDM Rx MIMO/Packet_Detection/Internal PktDet (Sim Only!)/Sliding Window ==
  In : Inport  OutDataType=sfix(16)  OutScaling=2^0
  Accum1 : xbsIndex_r4/Accumulator  operation=Add  n_bits=22  overflow=Flag as error  scale=1  rst=off  hasbypass=off  en=off  dbl_ovrd=off  use_behavioral_HDL=on  implementation=Fabric  block_type=accum
      settings: infoedit="Adder or subtractor-based accumulator.   Output type and binary point position match the input.<P><P>Hardware notes: When \"Reinitialize with input 'b' on reset\" is selected, the accumulator is forced to run at the system rate even if the input 'b' is running at a slower rate."  xl_area=[12 23 0 22 0 0 0]  block_version=VER_STRING_GOES_HERE
  AddSub1 : xbsIndex_r4/AddSub  mode=Subtraction  use_carryin=off  use_carryout=off  en=off  latency=1  precision=Full  arith_type="Signed  (2's comp)"  n_bits=36  bin_pt=28  quantization=Truncate  overflow=Saturate  dbl_ovrd=off  use_behavioral_HDL=on  pipelined=off  use_rpm=off  hw_selection=Fabric  block_type=addsub
      settings: xl_area=[9 18 0 17 0 0 0]  block_version=VER_STRING_GOES_HERE
  Delay6 : xbsIndex_r4/Delay  en=off  latency=16  dbl_ovrd=off  reg_retiming=off  block_type=delay
      settings: infoedit=Hardware notes: A delay line is a chain, each link of which is an SRL16 followed by a flip-flop. If register retiming is enabled, the delay line is a chain of flip-flops.  xl_area=[9 17 0 17 0 0 0]  block_version=VER_STRING_GOES_HERE
  Out : Outport  OutDataType=sfix(16)  OutScaling=2^0
  wires:
  Accum1/1 -> Out/1
  In/1 -> Delay6/1, AddSub1/1
  AddSub1/1 -> Accum1/1
  Delay6/1 -> AddSub1/2

== system ofdm_txrx_mimo/OFDM Rx MIMO/Packet_Detection/Internal PktDet (Sim Only!)/Sliding Window1 ==
  In : Inport  OutDataType=sfix(16)  OutScaling=2^0
  Accum1 : xbsIndex_r4/Accumulator  operation=Add  n_bits=22  overflow=Flag as error  scale=1  rst=off  hasbypass=off  en=off  dbl_ovrd=off  use_behavioral_HDL=on  implementation=Fabric  block_type=accum
      settings: infoedit="Adder or subtractor-based accumulator.   Output type and binary point position match the input.<P><P>Hardware notes: When \"Reinitialize with input 'b' on reset\" is selected, the accumulator is forced to run at the system rate even if the input 'b' is running at a slower rate."  xl_area=[12 23 0 22 0 0 0]  block_version=VER_STRING_GOES_HERE
  AddSub1 : xbsIndex_r4/AddSub  mode=Subtraction  use_carryin=off  use_carryout=off  en=off  latency=1  precision=Full  arith_type="Signed  (2's comp)"  n_bits=36  bin_pt=28  quantization=Truncate  overflow=Saturate  dbl_ovrd=off  use_behavioral_HDL=on  pipelined=off  use_rpm=off  hw_selection=Fabric  block_type=addsub
      settings: xl_area=[9 18 0 17 0 0 0]  block_version=VER_STRING_GOES_HERE
  Delay6 : xbsIndex_r4/Delay  en=off  latency=16  dbl_ovrd=off  reg_retiming=off  block_type=delay
      settings: infoedit=Hardware notes: A delay line is a chain, each link of which is an SRL16 followed by a flip-flop. If register retiming is enabled, the delay line is a chain of flip-flops.  xl_area=[9 17 0 17 0 0 0]  block_version=VER_STRING_GOES_HERE
  Out : Outport  OutDataType=sfix(16)  OutScaling=2^0
  wires:
  Delay6/1 -> AddSub1/2
  AddSub1/1 -> Accum1/1
  In/1 -> AddSub1/1, Delay6/1
  Accum1/1 -> Out/1
